simulink model "TosAeb"  (R2021a)
settings: Solver=FixedStepDiscrete  StartTime=0.0  StopTime=inf  FixedStep=0.01  RelTol=1e-3
undefined symbols (not defined in the file or in its folder): ACCModSts, AccSts1, AEB_ObjectMovingOrientation, AEB_ObjectMovingState, CN_BTCActive, CN_CurrentGearR, CN_CurrentGearR_DHT, CN_PTCActive, CN_VDCActive, CN_VSC_EspOff, CN_VSC_FCW_AEB_Error, CN_VSC_FCW_AEB_NoDispay, CN_VSC_FCW_AEB_PerfmDgdtn, CN_VSC_FCW_AEB_TempUnAvlbl, CN_VSC_VehStandstill, CNaccelAvoidMax, CNaccelAvoidMin, CNbrakePedalPress, CNcomfortStrLeftAccelMax, CNcomfortStrLeftAccelMin, CNcomfortStrRightAccelMax, CNcomfortStrRightAccelMin, CNDrvDoorOpen, CNDrvModeEco, CNDrvModeNorm, CNDrvModeOther, CNDrvModeSport, CNDrvSBRreminder, CNNull, CNobjInvalidID, CNPassDoorOpen, CNPassSBRreminder, CNPEBSAccelNegInf, CNPEBSAccelNull, CNPEBSTimeInf, CNPEBSTimeNull, CNSTOPMAX, CNSTOPNULL, CNsubjWidth, CNSwtOFF, CNSwtON, CNTTCMAX, CNTTCNULL, CNVehConfigAEBCycNone, CNVehConfigAEBNone, CNVehConfigAEBPedNone, CNVehConfigFCWNone, CNVehConfigJANone, CollisionSeverity, FALSE, FCWSnvty, JACycObjCalcInfoDefaultParams, JAPedObjCalcInfoDefaultParams, JAVehObjCalcInfoDefaultParams, latCycObjCalcInfoDefaultParams, latPedObjCalcInfoDefaultParams, longObjCalcInfoDefaultParams, ModSts, movingStatus, ObjectType ... (+1112)
libraries: simulink x3031, AEBSFunctionLib x50, Ta_Library x33, AEBSStateMachineLib x23, Mylibrary x12, simulink_extras x7, ObjSelect_lib x3, dspstat3 x2
(part 12 of 102)

== system TosAeb/FcwAebJa_Step_sys/ALG_AEBSystem/ALG_AEBOutput/AEB_EDR_JAPED_INFOMap/Subsystem ==
  JaPedTgtCalcInfo : Inport
  Action Port : ActionPort  ActionPortLabel=else
  Bus Creator : BusCreator  Inputs=25  OutDataTypeStr=Bus: AEB_EDR_LATPED_INFO_BUS
      settings: InheritFromInputs=off
  Bus Selector : BusSelector  OutputSignals=JAPedTgtObjInfo.AEBJAPedObj.FusionType,JAPedTgtObjInfo.AEBJAPedObj.ObjectID,JAPedTgtObjInfo.AEBJAPedObj.ObjectType,JAPedTgtObjInfo.AEBJAPedObj.ObjectTypeCameraConfirmed,JAPedTgtObjInfo.AEBJAPedObj.ObjectLength,JAPedTgtObjInfo.AEBJAPedObj.ObjectWidth,JAPedTgtObjInfo.AEBJAPedObj.ObjectHight,JAPedTgtObjInfo.AEBJAPedObj.ObjectYawAngle,JAPedTgtObjInfo.AEBJAPedObj.ObjectMovingState,JAPedTgtObjInfo.AEBJAPedObj.ObjectMovingOrientation,JAPedTgtObjInfo.AEBJAPedObj.dxFisrtDetection,JAPedTgtObjInfo.AEBJAPedObj.dyFisrtDetection,JAPedTgtObjInfo.AEBJAPedObj.dx,JAPedTgtObjInfo.AEBJAPedObj.dy,JAPedTgtObjInfo.AEBJAPedObj.dz,JAPedTgtObjInfo.AEBJAPedObj.vx,JAPedTgtObjInfo.AEBJAPedObj.vy,JAPedTgtObjInfo.AEBJAPedObj.ax,JAPedTgtObjInfo.AEBJAPedObj.ay,JAPedTgtObjInfo.AEBJAPedObj.dxVariance,JAPedTgtObjInfo.AEBJAPedObj.dyVariance,JAPedTgtObjInfo.AEBJAPedObj.ObjectExistProb,JAPedTgtObjInfo.AEBJAPedObj.ObjectIsObstacleProb,JAPedTgtObjInfo.AEBJAPedObj.ObjectMeasurementStatus,JAPedTgtObjInfo.AEBJAPedObj.ObjectLifeCycle
  Data Type Conversion{1..4} (4 alike) : DataTypeConversion  OutDataTypeStr=uint8
  Signal Conversion{,1..24} (25 alike) : SignalConversion  OverrideOpt=off
  AEB_EDR_JAPED_INFO : Outport  OutDataTypeStr=Bus: AEB_EDR_JAPED_INFO_BUS
  wires:
  Bus Selector/9 -> Signal Conversion8/1  "<ObjectMovingState>"
  Signal Conversion23/1 -> Bus Creator/24  "ObjectMeasurementStatus"
  Signal Conversion20/1 -> Bus Creator/11  "dxFisrtDetection"
  Bus Selector/18 -> Signal Conversion12/1  "<ax>"
  Bus Selector/23 -> Signal Conversion22/1  "<ObjectIsObstacleProb>"
  Signal Conversion13/1 -> Bus Creator/19  "ay"
  Bus Selector/25 -> Signal Conversion19/1  "<ObjectLifeCycle>"
  Bus Selector/1 -> Signal Conversion/1  "<FusionType>"
  Bus Selector/16 -> Signal Conversion15/1  "<vx>"
  Signal Conversion21/1 -> Bus Creator/22  "ObjectExistProb"
  JaPedTgtCalcInfo/1 -> Bus Selector/1
  Signal Conversion22/1 -> Bus Creator/23  "ObjectIsObstacleProb"
  Bus Selector/11 -> Signal Conversion20/1  "<dxFisrtDetection>"
  Signal Conversion10/1 -> Bus Creator/13  "dx"
  Signal Conversion4/1 -> Bus Creator/5  "ObjectLength"
  Bus Selector/7 -> Signal Conversion6/1  "<ObjectHight>"
  Bus Selector/10 -> Signal Conversion9/1  "<ObjectMovingOrientation>"
  Signal Conversion16/1 -> Bus Creator/17  "vy"
  Bus Selector/4 -> Signal Conversion3/1  "<ObjectTypeCameraConfirmed>"
  Bus Selector/5 -> Signal Conversion4/1  "<ObjectLength>"
  Bus Selector/14 -> Signal Conversion11/1  "<dy>"
  Signal Conversion1/1 -> Bus Creator/2  "ObjectID"
  Bus Selector/2 -> Signal Conversion1/1  "<ObjectID>"
  Bus Selector/19 -> Signal Conversion13/1  "<ay>"
  Signal Conversion5/1 -> Bus Creator/6  "ObjectWidth"
  Bus Selector/24 -> Signal Conversion23/1  "<ObjectMeasurementStatus>"
  Data Type Conversion1/1 -> Bus Creator/10  "ObjectMovingOrientation"
  Data Type Conversion4/1 -> Bus Creator/9  "ObjectMovingState"
  Bus Creator/1 -> AEB_EDR_JAPED_INFO/1
  Signal Conversion7/1 -> Bus Creator/8  "ObjectYawAngle"
  Bus Selector/12 -> Signal Conversion24/1  "<dyFisrtDetection>"
  Signal Conversion6/1 -> Bus Creator/7  "ObjectHight"
  Signal Conversion12/1 -> Bus Creator/18  "ax"
  Data Type Conversion2/1 -> Bus Creator/1  "FusionType"
  Bus Selector/3 -> Signal Conversion2/1  "<ObjectType>"
  Signal Conversion18/1 -> Bus Creator/21  "dyVariance"
  Signal Conversion11/1 -> Bus Creator/14  "dy"
  Signal Conversion9/1 -> Data Type Conversion1/1
  Bus Selector/8 -> Signal Conversion7/1  "<ObjectYawAngle>"
  Bus Selector/21 -> Signal Conversion18/1  "<dyVariance>"
  Data Type Conversion3/1 -> Bus Creator/3  "ObjectType"
  Signal Conversion2/1 -> Data Type Conversion3/1
  Signal Conversion15/1 -> Bus Creator/16  "vx"
  Signal Conversion3/1 -> Bus Creator/4  "ObjectTypeCameraConfirmed"
  Bus Selector/6 -> Signal Conversion5/1  "<ObjectWidth>"
  Signal Conversion24/1 -> Bus Creator/12  "dyFisrtDetection"
  Signal Conversion14/1 -> Bus Creator/15  "dz"
  Signal Conversion8/1 -> Data Type Conversion4/1
  Bus Selector/22 -> Signal Conversion21/1  "<ObjectExistProb>"
  Bus Selector/15 -> Signal Conversion14/1  "<dz>"
  Bus Selector/13 -> Signal Conversion10/1  "<dx>"
  Signal Conversion17/1 -> Bus Creator/20  "dxVariance"
  Signal Conversion19/1 -> Bus Creator/25  "ObjectLifeCycle"
  Bus Selector/17 -> Signal Conversion16/1  "<vy>"
  Signal Conversion/1 -> Data Type Conversion2/1
  Bus Selector/20 -> Signal Conversion17/1  "<dxVariance>"

== system TosAeb/FcwAebJa_Step_sys/ALG_AEBSystem/ALG_AEBOutput/AEB_EDR_JAVEH_INFOMap/If Action Subsystem ==
  Action Port : ActionPort  ActionPortLabel=if(u1)
  Bus Creator : BusCreator  Inputs=25  OutDataTypeStr=Bus: AEB_EDR_LATPED_INFO_BUS
      settings: InheritFromInputs=off
  Constant{,1..24} (25 alike) : Constant  Value=0
  Data Type Conversion{,5..24} (21 alike) : DataTypeConversion
  Data Type Conversion{1..4} (4 alike) : DataTypeConversion  OutDataTypeStr=uint8
  AEB_EDR_JAVEH_INFO : Outport  OutDataTypeStr=Bus: AEB_EDR_JAVEH_INFO_BUS
  wires:
  Constant24/1 -> Data Type Conversion24/1
  Constant18/1 -> Data Type Conversion20/1
  Constant19/1 -> Data Type Conversion21/1
  Constant21/1 -> Data Type Conversion23/1
  Constant20/1 -> Data Type Conversion22/1
  Constant16/1 -> Data Type Conversion18/1
  Constant17/1 -> Data Type Conversion19/1
  Constant23/1 -> Data Type Conversion17/1
  Constant22/1 -> Data Type Conversion16/1
  Constant8/1 -> Data Type Conversion4/1
  Constant9/1 -> Data Type Conversion1/1
  Constant11/1 -> Data Type Conversion11/1
  Constant10/1 -> Data Type Conversion10/1
  Constant14/1 -> Data Type Conversion14/1
  Constant15/1 -> Data Type Conversion15/1
  Constant13/1 -> Data Type Conversion13/1
  Constant12/1 -> Data Type Conversion12/1
  Constant4/1 -> Data Type Conversion6/1
  Constant5/1 -> Data Type Conversion7/1
  Constant7/1 -> Data Type Conversion9/1
  Constant6/1 -> Data Type Conversion8/1
  Constant2/1 -> Data Type Conversion3/1
  Constant3/1 -> Data Type Conversion5/1
  Constant1/1 -> Data Type Conversion/1
  Constant/1 -> Data Type Conversion2/1
  Bus Creator/1 -> AEB_EDR_JAVEH_INFO/1
  Data Type Conversion16/1 -> Bus Creator/17  "vy"
  Data Type Conversion9/1 -> Bus Creator/8  "ObjectYawAngle"
  Data Type Conversion22/1 -> Bus Creator/23  "ObjectIsObstacleProb"
  Data Type Conversion17/1 -> Bus Creator/18  "ax"
  Data Type Conversion11/1 -> Bus Creator/12  "dyFisrtDetection"
  Data Type Conversion19/1 -> Bus Creator/20  "dxVariance"
  Data Type Conversion15/1 -> Bus Creator/16  "vx"
  Data Type Conversion8/1 -> Bus Creator/7  "ObjectHight"
  Data Type Conversion14/1 -> Bus Creator/15  "dz"
  Data Type Conversion/1 -> Bus Creator/2  "ObjectID"
  Data Type Conversion4/1 -> Bus Creator/9  "ObjectMovingState"
  Data Type Conversion21/1 -> Bus Creator/22  "ObjectExistProb"
  Data Type Conversion3/1 -> Bus Creator/3  "ObjectType"
  Data Type Conversion1/1 -> Bus Creator/10  "ObjectMovingOrientation"
  Data Type Conversion24/1 -> Bus Creator/25  "ObjectLifeCycle"
  Data Type Conversion18/1 -> Bus Creator/19  "ay"
  Data Type Conversion6/1 -> Bus Creator/5  "ObjectLength"
  Data Type Conversion20/1 -> Bus Creator/21  "dyVariance"
  Data Type Conversion12/1 -> Bus Creator/13  "dx"
  Data Type Conversion2/1 -> Bus Creator/1  "FusionType"
  Data Type Conversion5/1 -> Bus Creator/4  "ObjectTypeCameraConfirmed"
  Data Type Conversion23/1 -> Bus Creator/24  "ObjectMeasurementStatus"
  Data Type Conversion10/1 -> Bus Creator/11  "dxFisrtDetection"
  Data Type Conversion13/1 -> Bus Creator/14  "dy"
  Data Type Conversion7/1 -> Bus Creator/6  "ObjectWidth"

== system TosAeb/FcwAebJa_Step_sys/ALG_AEBSystem/ALG_AEBOutput/AEB_EDR_JAVEH_INFOMap/Subsystem ==
  JaVehTgtCalcInfo : Inport
  Action Port : ActionPort  ActionPortLabel=else
  Bus Creator : BusCreator  Inputs=25  OutDataTypeStr=Bus: AEB_EDR_LATPED_INFO_BUS
      settings: InheritFromInputs=off
  Bus Selector : BusSelector  OutputSignals=JAVehTgtObjInfo.AEBJAVehObj.FusionType,JAVehTgtObjInfo.AEBJAVehObj.ObjectID,JAVehTgtObjInfo.AEBJAVehObj.ObjectType,JAVehTgtObjInfo.AEBJAVehObj.ObjectTypeCameraConfirmed,JAVehTgtObjInfo.AEBJAVehObj.ObjectLength,JAVehTgtObjInfo.AEBJAVehObj.ObjectWidth,JAVehTgtObjInfo.AEBJAVehObj.ObjectHight,JAVehTgtObjInfo.AEBJAVehObj.ObjectYawAngle,JAVehTgtObjInfo.AEBJAVehObj.ObjectMovingState,JAVehTgtObjInfo.AEBJAVehObj.ObjectMovingOrientation,JAVehTgtObjInfo.AEBJAVehObj.dxFisrtDetection,JAVehTgtObjInfo.AEBJAVehObj.dyFisrtDetection,JAVehTgtObjInfo.AEBJAVehObj.dx,JAVehTgtObjInfo.AEBJAVehObj.dy,JAVehTgtObjInfo.AEBJAVehObj.dz,JAVehTgtObjInfo.AEBJAVehObj.vx,JAVehTgtObjInfo.AEBJAVehObj.vy,JAVehTgtObjInfo.AEBJAVehObj.ax,JAVehTgtObjInfo.AEBJAVehObj.ay,JAVehTgtObjInfo.AEBJAVehObj.dxVariance,JAVehTgtObjInfo.AEBJAVehObj.dyVariance,JAVehTgtObjInfo.AEBJAVehObj.ObjectExistProb,JAVehTgtObjInfo.AEBJAVehObj.ObjectIsObstacleProb,JAVehTgtObjInfo.AEBJAVehObj.ObjectMeasurementStatus,JAVehTgtObjInfo.AEBJAVehObj.ObjectLifeCycle
  Data Type Conversion{1..4} (4 alike) : DataTypeConversion  OutDataTypeStr=uint8
  Signal Conversion{,1..24} (25 alike) : SignalConversion  OverrideOpt=off
  AEB_EDR_JAVEH_INFO : Outport  OutDataTypeStr=Bus: AEB_EDR_JAVEH_INFO_BUS
  wires:
  Bus Creator/1 -> AEB_EDR_JAVEH_INFO/1
  Bus Selector/18 -> Signal Conversion12/1  "<ax>"
  Bus Selector/21 -> Signal Conversion18/1  "<dyVariance>"
  Bus Selector/1 -> Signal Conversion/1  "<FusionType>"
  Signal Conversion20/1 -> Bus Creator/11  "dxFisrtDetection"
  Signal Conversion23/1 -> Bus Creator/24  "ObjectMeasurementStatus"
  Bus Selector/13 -> Signal Conversion10/1  "<dx>"
  Signal Conversion11/1 -> Bus Creator/14  "dy"
  Bus Selector/8 -> Signal Conversion7/1  "<ObjectYawAngle>"
  Bus Selector/19 -> Signal Conversion13/1  "<ay>"
  Signal Conversion3/1 -> Bus Creator/4  "ObjectTypeCameraConfirmed"
  Bus Selector/22 -> Signal Conversion21/1  "<ObjectExistProb>"
  Bus Selector/20 -> Signal Conversion17/1  "<dxVariance>"
  Signal Conversion5/1 -> Bus Creator/6  "ObjectWidth"
  Signal Conversion17/1 -> Bus Creator/20  "dxVariance"
  Signal Conversion15/1 -> Bus Creator/16  "vx"
  Signal Conversion7/1 -> Bus Creator/8  "ObjectYawAngle"
  Signal Conversion12/1 -> Bus Creator/18  "ax"
  Signal Conversion2/1 -> Data Type Conversion3/1
  Bus Selector/3 -> Signal Conversion2/1  "<ObjectType>"
  Bus Selector/14 -> Signal Conversion11/1  "<dy>"
  JaVehTgtCalcInfo/1 -> Bus Selector/1
  Bus Selector/11 -> Signal Conversion20/1  "<dxFisrtDetection>"
  Data Type Conversion2/1 -> Bus Creator/1  "FusionType"
  Bus Selector/16 -> Signal Conversion15/1  "<vx>"
  Signal Conversion10/1 -> Bus Creator/13  "dx"
  Signal Conversion18/1 -> Bus Creator/21  "dyVariance"
  Signal Conversion4/1 -> Bus Creator/5  "ObjectLength"
  Signal Conversion13/1 -> Bus Creator/19  "ay"
  Bus Selector/24 -> Signal Conversion23/1  "<ObjectMeasurementStatus>"
  Bus Selector/9 -> Signal Conversion8/1  "<ObjectMovingState>"
  Signal Conversion19/1 -> Bus Creator/25  "ObjectLifeCycle"
  Bus Selector/10 -> Signal Conversion9/1  "<ObjectMovingOrientation>"
  Data Type Conversion1/1 -> Bus Creator/10  "ObjectMovingOrientation"
  Bus Selector/2 -> Signal Conversion1/1  "<ObjectID>"
  Data Type Conversion3/1 -> Bus Creator/3  "ObjectType"
  Data Type Conversion4/1 -> Bus Creator/9  "ObjectMovingState"
  Bus Selector/12 -> Signal Conversion24/1  "<dyFisrtDetection>"
  Signal Conversion21/1 -> Bus Creator/22  "ObjectExistProb"
  Signal Conversion9/1 -> Data Type Conversion1/1
  Bus Selector/4 -> Signal Conversion3/1  "<ObjectTypeCameraConfirmed>"
  Bus Selector/5 -> Signal Conversion4/1  "<ObjectLength>"
  Signal Conversion14/1 -> Bus Creator/15  "dz"
  Signal Conversion1/1 -> Bus Creator/2  "ObjectID"
  Signal Conversion/1 -> Data Type Conversion2/1
  Signal Conversion22/1 -> Bus Creator/23  "ObjectIsObstacleProb"
  Bus Selector/23 -> Signal Conversion22/1  "<ObjectIsObstacleProb>"
  Signal Conversion8/1 -> Data Type Conversion4/1
  Bus Selector/7 -> Signal Conversion6/1  "<ObjectHight>"
  Bus Selector/15 -> Signal Conversion14/1  "<dz>"
  Bus Selector/6 -> Signal Conversion5/1  "<ObjectWidth>"
  Bus Selector/17 -> Signal Conversion16/1  "<vy>"
  Signal Conversion24/1 -> Bus Creator/12  "dyFisrtDetection"
  Signal Conversion16/1 -> Bus Creator/17  "vy"
  Signal Conversion6/1 -> Bus Creator/7  "ObjectHight"
  Bus Selector/25 -> Signal Conversion19/1  "<ObjectLifeCycle>"

== system TosAeb/FcwAebJa_Step_sys/ALG_AEBSystem/ALG_AEBOutput/AEB_EDR_LATCYC_INFOMap/If Action Subsystem ==
  Action Port : ActionPort  ActionPortLabel=if(u1)
  Bus Creator : BusCreator  Inputs=25  OutDataTypeStr=Bus: AEB_EDR_LATPED_INFO_BUS
      settings: InheritFromInputs=off
  Constant{,1..17,24..30} (25 alike) : Constant  Value=0
  Data Type Conversion{,1..3} (4 alike) : DataTypeConversion  OutDataTypeStr=uint8
  Data Type Conversion{4..24} (21 alike) : DataTypeConversion
  AEB_EDR_LATCYC_INFO : Outport  OutDataTypeStr=Bus: AEB_EDR_LATCYC_INFO_BUS
  wires:
  Constant16/1 -> Data Type Conversion16/1
  Constant17/1 -> Data Type Conversion17/1
  Constant25/1 -> Data Type Conversion19/1
  Constant24/1 -> Data Type Conversion18/1
  Constant28/1 -> Data Type Conversion22/1
  Constant29/1 -> Data Type Conversion23/1
  Constant27/1 -> Data Type Conversion21/1
  Constant26/1 -> Data Type Conversion20/1
  Constant30/1 -> Data Type Conversion24/1
  Constant8/1 -> Data Type Conversion/1
  Constant9/1 -> Data Type Conversion1/1
  Constant11/1 -> Data Type Conversion11/1
  Constant10/1 -> Data Type Conversion10/1
  Constant14/1 -> Data Type Conversion14/1
  Constant15/1 -> Data Type Conversion15/1
  Constant13/1 -> Data Type Conversion13/1
  Constant12/1 -> Data Type Conversion12/1
  Constant4/1 -> Data Type Conversion6/1
  Constant5/1 -> Data Type Conversion7/1
  Constant7/1 -> Data Type Conversion9/1
  Constant6/1 -> Data Type Conversion8/1
  Constant2/1 -> Data Type Conversion3/1
  Constant3/1 -> Data Type Conversion5/1
  Constant1/1 -> Data Type Conversion4/1
  Constant/1 -> Data Type Conversion2/1
  Data Type Conversion17/1 -> Bus Creator/18  "ax"
  Data Type Conversion23/1 -> Bus Creator/24  "ObjectMeasurementStatus"
  Data Type Conversion19/1 -> Bus Creator/20  "dxVariance"
  Data Type Conversion9/1 -> Bus Creator/8  "ObjectYawAngle"
  Data Type Conversion/1 -> Bus Creator/9  "ObjectMovingState"
  Data Type Conversion15/1 -> Bus Creator/16  "vx"
  Bus Creator/1 -> AEB_EDR_LATCYC_INFO/1
  Data Type Conversion6/1 -> Bus Creator/5  "ObjectLength"
  Data Type Conversion10/1 -> Bus Creator/11  "dxFisrtDetection"
  Data Type Conversion18/1 -> Bus Creator/19  "ay"
  Data Type Conversion12/1 -> Bus Creator/13  "dx"
  Data Type Conversion7/1 -> Bus Creator/6  "ObjectWidth"
  Data Type Conversion8/1 -> Bus Creator/7  "ObjectHight"
  Data Type Conversion11/1 -> Bus Creator/12  "dyFisrtDetection"
  Data Type Conversion16/1 -> Bus Creator/17  "vy"
  Data Type Conversion20/1 -> Bus Creator/21  "dyVariance"
  Data Type Conversion1/1 -> Bus Creator/10  "ObjectMovingOrientation"
  Data Type Conversion5/1 -> Bus Creator/4  "ObjectTypeCameraConfirmed"
  Data Type Conversion13/1 -> Bus Creator/14  "dy"
  Data Type Conversion22/1 -> Bus Creator/23  "ObjectIsObstacleProb"
  Data Type Conversion4/1 -> Bus Creator/2  "ObjectID"
  Data Type Conversion21/1 -> Bus Creator/22  "ObjectExistProb"
  Data Type Conversion24/1 -> Bus Creator/25  "ObjectLifeCycle"
  Data Type Conversion14/1 -> Bus Creator/15  "dz"
  Data Type Conversion2/1 -> Bus Creator/1  "FusionType"
  Data Type Conversion3/1 -> Bus Creator/3  "ObjectType"

== system TosAeb/FcwAebJa_Step_sys/ALG_AEBSystem/ALG_AEBOutput/AEB_EDR_LATCYC_INFOMap/Subsystem ==
  latCycTgtCalcInfo : Inport
  Action Port : ActionPort  ActionPortLabel=else
  Bus Creator : BusCreator  Inputs=25  OutDataTypeStr=Bus: AEB_EDR_LATPED_INFO_BUS
      settings: InheritFromInputs=off
  Bus Selector : BusSelector  OutputSignals=latCycObjCalcInfo.latCycObjRawInfo.FusionType,latCycObjCalcInfo.latCycObjRawInfo.ObjectID,latCycObjCalcInfo.latCycObjRawInfo.ObjectType,latCycObjCalcInfo.latCycObjRawInfo.ObjectTypeCameraConfirmed,latCycObjCalcInfo.latCycObjRawInfo.ObjectLength,latCycObjCalcInfo.latCycObjRawInfo.ObjectWidth,latCycObjCalcInfo.latCycObjRawInfo.ObjectHight,latCycObjCalcInfo.latCycObjRawInfo.ObjectYawAngle,latCycObjCalcInfo.latCycObjRawInfo.ObjectMovingState,latCycObjCalcInfo.latCycObjRawInfo.ObjectMovingOrientation,latCycObjCalcInfo.latCycObjRawInfo.dxFisrtDetection,latCycObjCalcInfo.latCycObjRawInfo.dyFisrtDetection,latCycObjCalcInfo.latCycObjRawInfo.dx,latCycObjCalcInfo.latCycObjRawInfo.dy,latCycObjCalcInfo.latCycObjRawInfo.dz,latCycObjCalcInfo.latCycObjRawInfo.vx,latCycObjCalcInfo.latCycObjRawInfo.vy,latCycObjCalcInfo.latCycObjRawInfo.ax,latCycObjCalcInfo.latCycObjRawInfo.ay,latCycObjCalcInfo.latCycObjRawInfo.dxVariance,latCycObjCalcInfo.latCycObjRawInfo.dyVariance,latCycObjCalcInfo.latCycObjRawInfo.ObjectExistProb,latCycObjCalcInfo.latCycObjRawInfo.ObjectIsObstacleProb,latCycObjCalcInfo.latCycObjRawInfo.ObjectMeasurementStatus,latCycObjCalcInfo.latCycObjRawInfo.ObjectLifeCycle
  Data Type Conversion{,1..3} (4 alike) : DataTypeConversion  OutDataTypeStr=uint8
  Signal Conversion{,1..24} (25 alike) : SignalConversion  OverrideOpt=off
  AEB_EDR_LATCYC_INFO : Outport  OutDataTypeStr=Bus: AEB_EDR_LATCYC_INFO_BUS
  wires:
  Data Type Conversion3/1 -> Bus Creator/3  "ObjectType"
  Data Type Conversion2/1 -> Bus Creator/1  "FusionType"
  Signal Conversion14/1 -> Bus Creator/15  "dz"
  Bus Selector/24 -> Signal Conversion23/1  "<ObjectMeasurementStatus>"
  Signal Conversion21/1 -> Bus Creator/22  "ObjectExistProb"
  Signal Conversion22/1 -> Bus Creator/23  "ObjectIsObstacleProb"
  Signal Conversion1/1 -> Bus Creator/2  "ObjectID"
  Signal Conversion11/1 -> Bus Creator/14  "dy"
  Bus Selector/22 -> Signal Conversion21/1  "<ObjectExistProb>"
  Signal Conversion3/1 -> Bus Creator/4  "ObjectTypeCameraConfirmed"
  Data Type Conversion1/1 -> Bus Creator/10  "ObjectMovingOrientation"
  Bus Selector/4 -> Signal Conversion3/1  "<ObjectTypeCameraConfirmed>"
  Bus Selector/25 -> Signal Conversion19/1  "<ObjectLifeCycle>"
  Bus Selector/12 -> Signal Conversion24/1  "<dyFisrtDetection>"
  Signal Conversion9/1 -> Data Type Conversion1/1
  Bus Selector/1 -> Signal Conversion/1  "<FusionType>"
  Bus Selector/19 -> Signal Conversion13/1  "<ay>"
  Signal Conversion18/1 -> Bus Creator/21  "dyVariance"
  Bus Selector/5 -> Signal Conversion4/1  "<ObjectLength>"
  Bus Selector/7 -> Signal Conversion6/1  "<ObjectHight>"
  Signal Conversion24/1 -> Bus Creator/12  "dyFisrtDetection"
  Signal Conversion6/1 -> Bus Creator/7  "ObjectHight"
  Bus Selector/2 -> Signal Conversion1/1  "<ObjectID>"
  Signal Conversion5/1 -> Bus Creator/6  "ObjectWidth"
  Signal Conversion13/1 -> Bus Creator/19  "ay"
  Signal Conversion10/1 -> Bus Creator/13  "dx"
  Signal Conversion4/1 -> Bus Creator/5  "ObjectLength"
  Signal Conversion19/1 -> Bus Creator/25  "ObjectLifeCycle"
  Bus Creator/1 -> AEB_EDR_LATCYC_INFO/1
  Bus Selector/8 -> Signal Conversion7/1  "<ObjectYawAngle>"
  Bus Selector/13 -> Signal Conversion10/1  "<dx>"
  Bus Selector/14 -> Signal Conversion11/1  "<dy>"
  Signal Conversion15/1 -> Bus Creator/16  "vx"
  Bus Selector/9 -> Signal Conversion8/1  "<ObjectMovingState>"
  Signal Conversion/1 -> Data Type Conversion2/1
  Signal Conversion8/1 -> Data Type Conversion/1
  Bus Selector/18 -> Signal Conversion12/1  "<ax>"
  Data Type Conversion/1 -> Bus Creator/9  "ObjectMovingState"
  Signal Conversion16/1 -> Bus Creator/17  "vy"
  Bus Selector/21 -> Signal Conversion18/1  "<dyVariance>"
  Signal Conversion7/1 -> Bus Creator/8  "ObjectYawAngle"
  Bus Selector/6 -> Signal Conversion5/1  "<ObjectWidth>"
  Signal Conversion17/1 -> Bus Creator/20  "dxVariance"
  Bus Selector/23 -> Signal Conversion22/1  "<ObjectIsObstacleProb>"
  Bus Selector/10 -> Signal Conversion9/1  "<ObjectMovingOrientation>"
  latCycTgtCalcInfo/1 -> Bus Selector/1
  Bus Selector/16 -> Signal Conversion15/1  "<vx>"
  Signal Conversion23/1 -> Bus Creator/24  "ObjectMeasurementStatus"
  Signal Conversion2/1 -> Data Type Conversion3/1
  Bus Selector/3 -> Signal Conversion2/1  "<ObjectType>"
  Signal Conversion20/1 -> Bus Creator/11  "dxFisrtDetection"
  Signal Conversion12/1 -> Bus Creator/18  "ax"
  Bus Selector/17 -> Signal Conversion16/1  "<vy>"
  Bus Selector/15 -> Signal Conversion14/1  "<dz>"
  Bus Selector/11 -> Signal Conversion20/1  "<dxFisrtDetection>"
  Bus Selector/20 -> Signal Conversion17/1  "<dxVariance>"

== system TosAeb/FcwAebJa_Step_sys/ALG_AEBSystem/ALG_AEBOutput/AEB_EDR_LATPED_INFOMap/If Action Subsystem ==
  Action Port : ActionPort  ActionPortLabel=if(u1)
  Bus Creator : BusCreator  Inputs=25  OutDataTypeStr=Bus: AEB_EDR_LATPED_INFO_BUS
      settings: InheritFromInputs=off
  Constant{,1..24} (25 alike) : Constant  Value=0
  Data Type Conversion{,1..3} (4 alike) : DataTypeConversion  OutDataTypeStr=uint8
  Data Type Conversion{4..24} (21 alike) : DataTypeConversion
  AEB_EDR_LATPED_INFO : Outport  OutDataTypeStr=Bus: AEB_EDR_LATPED_INFO_BUS
  wires:
  Constant23/1 -> Data Type Conversion21/1
  Constant22/1 -> Data Type Conversion24/1
  Constant24/1 -> Data Type Conversion23/1
  Constant21/1 -> Data Type Conversion18/1
  Constant20/1 -> Data Type Conversion19/1
  Constant18/1 -> Data Type Conversion20/1
  Constant19/1 -> Data Type Conversion17/1
  Constant11/1 -> Data Type Conversion10/1
  Constant10/1 -> Data Type Conversion9/1
  Constant12/1 -> Data Type Conversion12/1
  Constant13/1 -> Data Type Conversion11/1
  Constant17/1 -> Data Type Conversion14/1
  Constant16/1 -> Data Type Conversion15/1
  Constant14/1 -> Data Type Conversion16/1
  Constant15/1 -> Data Type Conversion13/1
  Constant7/1 -> Data Type Conversion22/1
  Constant6/1 -> Data Type Conversion7/1
  Constant8/1 -> Data Type Conversion/1
  Constant9/1 -> Data Type Conversion1/1
  Constant5/1 -> Data Type Conversion8/1
  Constant4/1 -> Data Type Conversion6/1
  Constant2/1 -> Data Type Conversion3/1
  Constant3/1 -> Data Type Conversion5/1
  Constant1/1 -> Data Type Conversion4/1
  Data Type Conversion23/1 -> Bus Creator/25  "ObjectLifeCycle"
  Data Type Conversion19/1 -> Bus Creator/21  "dyVariance"
  Bus Creator/1 -> AEB_EDR_LATPED_INFO/1
  Data Type Conversion6/1 -> Bus Creator/5  "ObjectLength"
  Data Type Conversion21/1 -> Bus Creator/24  "ObjectMeasurementStatus"
  Data Type Conversion17/1 -> Bus Creator/20  "dxVariance"
  Data Type Conversion13/1 -> Bus Creator/16  "vx"
  Data Type Conversion14/1 -> Bus Creator/18  "ax"
  Data Type Conversion4/1 -> Bus Creator/2  "ObjectID"
  Data Type Conversion1/1 -> Bus Creator/10  "ObjectMovingOrientation"
  Data Type Conversion/1 -> Bus Creator/9  "ObjectMovingState"
  Data Type Conversion18/1 -> Bus Creator/22  "ObjectExistProb"
  Data Type Conversion12/1 -> Bus Creator/13  "dx"
  Data Type Conversion16/1 -> Bus Creator/15  "dz"
  Data Type Conversion20/1 -> Bus Creator/19  "ay"
  Data Type Conversion7/1 -> Bus Creator/7  "ObjectHight"
  Data Type Conversion15/1 -> Bus Creator/17  "vy"
  Constant/1 -> Data Type Conversion2/1
  Data Type Conversion5/1 -> Bus Creator/4  "ObjectTypeCameraConfirmed"
  Data Type Conversion22/1 -> Bus Creator/8  "ObjectYawAngle"
  Data Type Conversion24/1 -> Bus Creator/23  "ObjectIsObstacleProb"
  Data Type Conversion8/1 -> Bus Creator/6  "ObjectWidth"
  Data Type Conversion11/1 -> Bus Creator/14  "dy"
  Data Type Conversion9/1 -> Bus Creator/11  "dxFisrtDetection"
  Data Type Conversion10/1 -> Bus Creator/12  "dyFisrtDetection"
  Data Type Conversion2/1 -> Bus Creator/1  "FusionType"
  Data Type Conversion3/1 -> Bus Creator/3  "ObjectType"

== system TosAeb/FcwAebJa_Step_sys/ALG_AEBSystem/ALG_AEBOutput/AEB_EDR_LATPED_INFOMap/Subsystem ==
  latPedTgtCalcInfo : Inport
  Action Port : ActionPort  ActionPortLabel=else
  Bus Creator : BusCreator  Inputs=25  OutDataTypeStr=Bus: AEB_EDR_LATPED_INFO_BUS
      settings: InheritFromInputs=off
  Bus Selector : BusSelector  OutputSignals=latPedObjCalcInfo.latPedObjRawInfo.FusionType,latPedObjCalcInfo.latPedObjRawInfo.ObjectID,latPedObjCalcInfo.latPedObjRawInfo.ObjectType,latPedObjCalcInfo.latPedObjRawInfo.ObjectTypeCameraConfirmed,latPedObjCalcInfo.latPedObjRawInfo.ObjectLength,latPedObjCalcInfo.latPedObjRawInfo.ObjectWidth,latPedObjCalcInfo.latPedObjRawInfo.ObjectHight,latPedObjCalcInfo.latPedObjRawInfo.ObjectYawAngle,latPedObjCalcInfo.latPedObjRawInfo.ObjectMovingState,latPedObjCalcInfo.latPedObjRawInfo.ObjectMovingOrientation,latPedObjCalcInfo.latPedObjRawInfo.dxFisrtDetection,latPedObjCalcInfo.latPedObjRawInfo.dyFisrtDetection,latPedObjCalcInfo.latPedObjRawInfo.dx,latPedObjCalcInfo.latPedObjRawInfo.dy,latPedObjCalcInfo.latPedObjRawInfo.dz,latPedObjCalcInfo.latPedObjRawInfo.vx,latPedObjCalcInfo.latPedObjRawInfo.vy,latPedObjCalcInfo.latPedObjRawInfo.ax,latPedObjCalcInfo.latPedObjRawInfo.ay,latPedObjCalcInfo.latPedObjRawInfo.dxVariance,latPedObjCalcInfo.latPedObjRawInfo.dyVariance,latPedObjCalcInfo.latPedObjRawInfo.ObjectExistProb,latPedObjCalcInfo.latPedObjRawInfo.ObjectIsObstacleProb,latPedObjCalcInfo.latPedObjRawInfo.ObjectMeasurementStatus,latPedObjCalcInfo.latPedObjRawInfo.ObjectLifeCycle
  Data Type Conversion{,1..3} (4 alike) : DataTypeConversion  OutDataTypeStr=uint8
  Signal Conversion{,1..24} (25 alike) : SignalConversion  OverrideOpt=off
  AEB_EDR_LATPED_INFO : Outport  OutDataTypeStr=Bus: AEB_EDR_LATPED_INFO_BUS
  wires:
  Bus Selector/5 -> Signal Conversion4/1  "<ObjectLength>"
  Signal Conversion24/1 -> Bus Creator/12  "dyFisrtDetection"
  Bus Selector/20 -> Signal Conversion17/1  "<dxVariance>"
  Bus Selector/18 -> Signal Conversion12/1  "<ax>"
  Bus Selector/19 -> Signal Conversion13/1  "<ay>"
  Bus Selector/10 -> Signal Conversion9/1  "<ObjectMovingOrientation>"
  Signal Conversion20/1 -> Bus Creator/11  "dxFisrtDetection"
  Data Type Conversion2/1 -> Bus Creator/1  "FusionType"
  Bus Selector/23 -> Signal Conversion22/1  "<ObjectIsObstacleProb>"
  Bus Selector/16 -> Signal Conversion15/1  "<vx>"
  Signal Conversion11/1 -> Bus Creator/14  "dy"
  Bus Selector/24 -> Signal Conversion23/1  "<ObjectMeasurementStatus>"
  Bus Selector/21 -> Signal Conversion18/1  "<dyVariance>"
  Signal Conversion22/1 -> Bus Creator/23  "ObjectIsObstacleProb"
  Signal Conversion5/1 -> Bus Creator/6  "ObjectWidth"
  Bus Selector/12 -> Signal Conversion24/1  "<dyFisrtDetection>"
  Bus Selector/11 -> Signal Conversion20/1  "<dxFisrtDetection>"
  Signal Conversion3/1 -> Bus Creator/4  "ObjectTypeCameraConfirmed"
  Bus Selector/25 -> Signal Conversion19/1  "<ObjectLifeCycle>"
  Signal Conversion9/1 -> Data Type Conversion1/1
  Signal Conversion6/1 -> Bus Creator/7  "ObjectHight"
  Bus Selector/7 -> Signal Conversion6/1  "<ObjectHight>"
  Signal Conversion10/1 -> Bus Creator/13  "dx"
  Signal Conversion13/1 -> Bus Creator/19  "ay"
  Bus Selector/3 -> Signal Conversion2/1  "<ObjectType>"
  Bus Selector/4 -> Signal Conversion3/1  "<ObjectTypeCameraConfirmed>"
  Signal Conversion14/1 -> Bus Creator/15  "dz"
  Bus Selector/8 -> Signal Conversion7/1  "<ObjectYawAngle>"
  Bus Selector/17 -> Signal Conversion16/1  "<vy>"
  Signal Conversion8/1 -> Data Type Conversion/1
  Bus Selector/2 -> Signal Conversion1/1  "<ObjectID>"
  Bus Selector/6 -> Signal Conversion5/1  "<ObjectWidth>"
  Signal Conversion21/1 -> Bus Creator/22  "ObjectExistProb"
  Data Type Conversion/1 -> Bus Creator/9  "ObjectMovingState"
  Data Type Conversion3/1 -> Bus Creator/3  "ObjectType"
  Data Type Conversion1/1 -> Bus Creator/10  "ObjectMovingOrientation"
  Signal Conversion7/1 -> Bus Creator/8  "ObjectYawAngle"
  Signal Conversion2/1 -> Data Type Conversion3/1
  Signal Conversion1/1 -> Bus Creator/2  "ObjectID"
  Bus Selector/13 -> Signal Conversion10/1  "<dx>"
  Signal Conversion15/1 -> Bus Creator/16  "vx"
  Bus Selector/1 -> Signal Conversion/1  "<FusionType>"
  Signal Conversion12/1 -> Bus Creator/18  "ax"
  Signal Conversion16/1 -> Bus Creator/17  "vy"
  Signal Conversion4/1 -> Bus Creator/5  "ObjectLength"
  Signal Conversion23/1 -> Bus Creator/24  "ObjectMeasurementStatus"
  Bus Creator/1 -> AEB_EDR_LATPED_INFO/1
  latPedTgtCalcInfo/1 -> Bus Selector/1
  Signal Conversion18/1 -> Bus Creator/21  "dyVariance"
  Signal Conversion19/1 -> Bus Creator/25  "ObjectLifeCycle"
  Bus Selector/15 -> Signal Conversion14/1  "<dz>"
  Bus Selector/9 -> Signal Conversion8/1  "<ObjectMovingState>"
  Bus Selector/14 -> Signal Conversion11/1  "<dy>"
  Signal Conversion17/1 -> Bus Creator/20  "dxVariance"
  Signal Conversion/1 -> Data Type Conversion2/1
  Bus Selector/22 -> Signal Conversion21/1  "<ObjectExistProb>"

== system TosAeb/FcwAebJa_Step_sys/ALG_AEBSystem/ALG_AEBOutput/AEB_EDR_LONOBJ_INFOMap/If Action Subsystem ==
  Action Port : ActionPort  ActionPortLabel=if(u1)
  Bus Creator : BusCreator  Inputs=24  OutDataTypeStr=Bus: AEB_EDR_LONOBJ_INFO_BUS
      settings: InheritFromInputs=off
  Constant{,1..23} (24 alike) : Constant  Value=0
  Data Type Conversion{,5..23} (20 alike) : DataTypeConversion
  Data Type Conversion{1..4} (4 alike) : DataTypeConversion  OutDataTypeStr=uint8
  AEB_EDR_LONOBJ_INFO : Outport  OutDataTypeStr=Bus: AEB_EDR_LONOBJ_INFO_BUS
  wires:
  Constant1/1 -> Data Type Conversion23/1  "ObjectID"
  Constant7/1 -> Data Type Conversion22/1  "ObjectYawAngle"
  Constant6/1 -> Data Type Conversion21/1  "ObjectHight"
  Constant5/1 -> Data Type Conversion20/1  "ObjectWidth"
  Constant4/1 -> Data Type Conversion14/1  "ObjectLength"
  Constant3/1 -> Data Type Conversion13/1  "ObjectTypeCameraConfirmed"
  Constant10/1 -> Data Type Conversion/1  "dx"
  Constant11/1 -> Data Type Conversion5/1  "dy"
  Constant12/1 -> Data Type Conversion6/1  "dz"
  Constant13/1 -> Data Type Conversion7/1  "vx"
  Constant14/1 -> Data Type Conversion8/1  "vy"
  Constant15/1 -> Data Type Conversion9/1  "ax"
  Constant16/1 -> Data Type Conversion10/1  "ay"
  Constant17/1 -> Data Type Conversion11/1  "dxVariance"
  Constant18/1 -> Data Type Conversion12/1  "dyVariance"
  Constant19/1 -> Data Type Conversion15/1  "ObjectExistProb"
  Constant20/1 -> Data Type Conversion16/1  "ObjectIsObstacleProb"
  Constant21/1 -> Data Type Conversion17/1  "ObjectMeasurementStatus"
  Constant22/1 -> Data Type Conversion18/1  "dyObject2Center"
  Constant23/1 -> Data Type Conversion19/1  "dyEgo2Center"
  Constant9/1 -> Data Type Conversion4/1  "ObjectID"
  Constant8/1 -> Data Type Conversion1/1
  Constant2/1 -> Data Type Conversion3/1
  Constant/1 -> Data Type Conversion2/1
  Data Type Conversion8/1 -> Bus Creator/15  "vy"
  Data Type Conversion5/1 -> Bus Creator/12  "dy"
  Data Type Conversion/1 -> Bus Creator/11  "dx"
  Data Type Conversion23/1 -> Bus Creator/2  "ObjectID"
  Data Type Conversion16/1 -> Bus Creator/21  "ObjectIsObstacleProb"
  Data Type Conversion6/1 -> Bus Creator/13  "dz"
  Data Type Conversion15/1 -> Bus Creator/20  "ObjectExistProb"
  Data Type Conversion12/1 -> Bus Creator/19  "dyVariance"
  Data Type Conversion7/1 -> Bus Creator/14  "vx"
  Data Type Conversion11/1 -> Bus Creator/18  "dxVariance"
  Data Type Conversion10/1 -> Bus Creator/17  "ay"
  Data Type Conversion21/1 -> Bus Creator/7  "ObjectHight"
  Data Type Conversion14/1 -> Bus Creator/5  "ObjectLength"
  Data Type Conversion1/1 -> Bus Creator/9  "ObjectMovingState"
  Data Type Conversion9/1 -> Bus Creator/16  "ax"
  Data Type Conversion4/1 -> Bus Creator/10  "ObjectMovingOrientation"
  Data Type Conversion13/1 -> Bus Creator/4  "ObjectTypeCameraConfirmed"
  Data Type Conversion22/1 -> Bus Creator/8  "ObjectYawAngle"
  Data Type Conversion20/1 -> Bus Creator/6  "ObjectWidth"
  Data Type Conversion19/1 -> Bus Creator/24  "dyEgo2Center"
  Data Type Conversion18/1 -> Bus Creator/23  "dyObject2Center"
  Data Type Conversion2/1 -> Bus Creator/1  "FusionType"
  Bus Creator/1 -> AEB_EDR_LONOBJ_INFO/1
  Data Type Conversion17/1 -> Bus Creator/22  "ObjectMeasurementStatus"
  Data Type Conversion3/1 -> Bus Creator/3  "ObjectType"

== system TosAeb/FcwAebJa_Step_sys/ALG_AEBSystem/ALG_AEBOutput/AEB_EDR_LONOBJ_INFOMap/Subsystem ==
  longObjCalcInfo : Inport
  Action Port : ActionPort  ActionPortLabel=else
  Bus Creator : BusCreator  Inputs=24  OutDataTypeStr=Bus: AEB_EDR_LONOBJ_INFO_BUS
      settings: InheritFromInputs=off
  Bus Selector : BusSelector  OutputSignals=LongObjRawInfo.FusionType,LongObjRawInfo.ObjectID,LongObjRawInfo.ObjectType,LongObjRawInfo.ObjectTypeCameraConfirmed,LongObjRawInfo.ObjectLength,LongObjRawInfo.ObjectWidth,LongObjRawInfo.ObjectHight,LongObjRawInfo.ObjectYawAngle,LongObjRawInfo.ObjectMovingState,LongObjRawInfo.ObjectMovingOrientation,LongObjRawInfo.dx,LongObjRawInfo.dy,LongObjRawInfo.dz,LongObjRawInfo.vx,LongObjRawInfo.vy,LongObjRawInfo.ax,LongObjRawInfo.ay,LongObjRawInfo.dxVariance,LongObjRawInfo.dyVariance,LongObjRawInfo.ObjectExistProb,LongObjRawInfo.ObjectIsObstacleProb,LongObjRawInfo.ObjectMeasurementStatus,LongObjRawInfo.dyObject2Center,LongObjRawInfo.dyEgo2Center
  Data Type Conversion{1..4} (4 alike) : DataTypeConversion  OutDataTypeStr=uint8
  Signal Conversion{,1..23} (24 alike) : SignalConversion  OverrideOpt=off
  AEB_EDR_LONOBJ_INFO : Outport  OutDataTypeStr=Bus: AEB_EDR_LONOBJ_INFO_BUS
  wires:
  Bus Selector/9 -> Signal Conversion8/1  "<ObjectMovingState>"
  Bus Selector/11 -> Signal Conversion10/1  "<dx>"
  Bus Selector/3 -> Signal Conversion2/1  "<ObjectType>"
  Data Type Conversion2/1 -> Bus Creator/1  "FusionType"
  Signal Conversion2/1 -> Data Type Conversion3/1
  Signal Conversion19/1 -> Bus Creator/24  "dyEgo2Center"
  Bus Creator/1 -> AEB_EDR_LONOBJ_INFO/1
  Signal Conversion7/1 -> Bus Creator/8  "ObjectYawAngle"
  Bus Selector/21 -> Signal Conversion22/1  "<ObjectIsObstacleProb>"
  Signal Conversion12/1 -> Bus Creator/16  "ax"
  Data Type Conversion1/1 -> Bus Creator/9  "ObjectMovingState"
  Signal Conversion6/1 -> Bus Creator/7  "ObjectHight"
  Bus Selector/24 -> Signal Conversion19/1  "<dyEgo2Center>"
  Data Type Conversion4/1 -> Bus Creator/10  "ObjectMovingOrientation"
  Bus Selector/23 -> Signal Conversion23/1  "<dyObject2Center>"
  Signal Conversion21/1 -> Bus Creator/20  "ObjectExistProb"
  Signal Conversion8/1 -> Data Type Conversion1/1
  Bus Selector/13 -> Signal Conversion14/1  "<dz>"
  Bus Selector/14 -> Signal Conversion15/1  "<vx>"
  Signal Conversion17/1 -> Bus Creator/18  "dxVariance"
  longObjCalcInfo/1 -> Bus Selector/1
  Data Type Conversion3/1 -> Bus Creator/3  "ObjectType"
  Signal Conversion1/1 -> Bus Creator/2  "ObjectID"
  Signal Conversion22/1 -> Bus Creator/21  "ObjectIsObstacleProb"
  Bus Selector/8 -> Signal Conversion7/1  "<ObjectYawAngle>"
  Bus Selector/17 -> Signal Conversion13/1  "<ay>"
  Bus Selector/12 -> Signal Conversion11/1  "<dy>"
  Bus Selector/20 -> Signal Conversion21/1  "<ObjectExistProb>"
  Signal Conversion4/1 -> Bus Creator/5  "ObjectLength"
  Bus Selector/1 -> Signal Conversion/1  "<FusionType>"
  Signal Conversion14/1 -> Bus Creator/13  "dz"
  Bus Selector/22 -> Signal Conversion20/1  "<ObjectMeasurementStatus>"
  Signal Conversion3/1 -> Bus Creator/4  "ObjectTypeCameraConfirmed"
  Signal Conversion11/1 -> Bus Creator/12  "dy"
  Signal Conversion13/1 -> Bus Creator/17  "ay"
  Signal Conversion18/1 -> Bus Creator/19  "dyVariance"
  Bus Selector/18 -> Signal Conversion17/1  "<dxVariance>"
  Signal Conversion10/1 -> Bus Creator/11  "dx"
  Bus Selector/6 -> Signal Conversion5/1  "<ObjectWidth>"
  Bus Selector/5 -> Signal Conversion4/1  "<ObjectLength>"
  Signal Conversion5/1 -> Bus Creator/6  "ObjectWidth"
  Signal Conversion9/1 -> Data Type Conversion4/1
  Signal Conversion16/1 -> Bus Creator/15  "vy"
  Signal Conversion23/1 -> Bus Creator/23  "dyObject2Center"
  Bus Selector/16 -> Signal Conversion12/1  "<ax>"
  Bus Selector/4 -> Signal Conversion3/1  "<ObjectTypeCameraConfirmed>"
  Bus Selector/19 -> Signal Conversion18/1  "<dyVariance>"
  Bus Selector/7 -> Signal Conversion6/1  "<ObjectHight>"
  Signal Conversion20/1 -> Bus Creator/22  "ObjectMeasurementStatus"
  Signal Conversion15/1 -> Bus Creator/14  "vx"
  Bus Selector/10 -> Signal Conversion9/1  "<ObjectMovingOrientation>"
  Bus Selector/15 -> Signal Conversion16/1  "<vy>"
  Signal Conversion/1 -> Data Type Conversion2/1
  Bus Selector/2 -> Signal Conversion1/1  "<ObjectID>"

== system TosAeb/AEB_Objsel/TA_InfoRebuild/TAObjInfoCalc/For Each Subsystem/Info_rebuild/Basic_Info ==
  Obj_Infomation : Inport
  Abs : Abs
  Atan : Trigonometry  Operator=atan
  Bus Selector : BusSelector  OutputSignals=VelX_s,VelY_s,AccX_s,AccY_s,PosX_s,PosY_s
  Compare To Constant : simulink/Logic and Bit Operations/Compare To Constant  relop=<=  const=0.001  OutDataTypeStr=boolean
  Constant{,1..3,5} (5 alike) : Constant  Value=0  OutDataTypeStr=single
  Constant4 : Constant  Value=0.001  OutDataTypeStr=single
  Divide : Product  Inputs=*/
  Switch : Switch  Criteria=u2 > Threshold
  vxRel : Outport
  vyRel : Outport  Port=2
  vxRelStd : Outport  Port=3
  vyRelStd : Outport  Port=4
  axRel : Outport  Port=5
  ayRel : Outport  Port=6
  axRelStd : Outport  Port=7
  ayRelStd : Outport  Port=8
  azimuthAngle : Outport  Port=9
  azimuthAngleStd : Outport  Port=10
  wires:
  Obj_Infomation/1 -> Bus Selector/1
  Bus Selector/1 -> [VelX]  "<VelX_s>"
  Bus Selector/2 -> [VelY]  "<VelY_s>"
  Bus Selector/3 -> [AccX]  "<AccX_s>"
  Bus Selector/4 -> [AccY]  "<AccY_s>"
  Bus Selector/5 -> [PosX]  "<PosX_s>"
  Bus Selector/6 -> [PosY]  "<PosY_s>"
  [VelX] -> vxRel/1
  [VelY] -> vyRel/1
  Constant/1 -> vxRelStd/1
  Constant1/1 -> vyRelStd/1
  [AccX] -> axRel/1
  [AccY] -> ayRel/1
  Constant2/1 -> axRelStd/1
  Constant3/1 -> ayRelStd/1
  [PosX] -> Switch/3, Abs/1
  Compare To Constant/1 -> Switch/2
  Constant4/1 -> Switch/1
  Abs/1 -> Compare To Constant/1
  [PosY] -> Divide/1
  Switch/1 -> Divide/2
  Divide/1 -> Atan/1
  Atan/1 -> azimuthAngle/1
  Constant5/1 -> azimuthAngleStd/1

== system TosAeb/AEB_Objsel/TA_InfoRebuild/TAObjInfoCalc/For Each Subsystem/Info_rebuild/Road_relative ==
  Obj_Infomation : Inport
  Road_Info : Inport  Port=2
  LineInfo_Process : Subsystem
  RebuildInfo_Process : Subsystem
  objLatDist2LaneCenter : Outport
  objLatDist2EgoCenter : Outport  Port=2
  objPosition : Outport  Port=3
  wires:
  Road_Info/1 -> LineInfo_Process/1(Road_Info)
  Obj_Infomation/1 -> RebuildInfo_Process/1(Obj_Infomation)
  LineInfo_Process/1(LeftLine_Info) -> RebuildInfo_Process/2(LeftLine_Info)
  LineInfo_Process/2(RightLine_Info) -> RebuildInfo_Process/3(RightLine_Info)
  RebuildInfo_Process/2(objLatDist2EgoCenter) -> objLatDist2EgoCenter/1
  RebuildInfo_Process/1(objLatDist2LaneCenter) -> objLatDist2LaneCenter/1
  RebuildInfo_Process/3(objPosition) -> objPosition/1

== system TosAeb/AEB_Objsel/TA_Processor/AEB_ObjSelect/TargetInfoCheck/Data_precess/ObjInfo_Assignment ==
  Overlap_Flg : Inport
  Boudingbox_Calculate : Inport  Port=2
  Obj_Coef : Inport  Port=3
  TTI : Inport  Port=4
  LatObj_Valid : Inport  Port=5
  Lat_ObjIntersect : Inport  Port=6
  Lat_TTR : Inport  Port=7
  JA_TTR : Inport  Port=8
  Signal Conversion{,1..7} (8 alike) : SignalConversion  OverrideOpt=off
  Boudingbox_CalculateAssign : Outport
  Obj_CoefAssign : Outport  Port=2
  TTIAssign : Outport  Port=3
  LatObj_ValidAssign : Outport  Port=4
  Lat_ObjIntersectAssign : Outport  Port=5
  Lat_TTRAssign : Outport  Port=6
  Overlap_FlgAssign : Outport  Port=7
  JA_TTRAssign : Outport  Port=8
  wires:
  Signal Conversion6/1 -> Lat_TTRAssign/1
  Signal Conversion/1 -> Overlap_FlgAssign/1
  Signal Conversion4/1 -> LatObj_ValidAssign/1
  Signal Conversion1/1 -> Boudingbox_CalculateAssign/1
  Signal Conversion5/1 -> Lat_ObjIntersectAssign/1
  Signal Conversion2/1 -> Obj_CoefAssign/1
  Signal Conversion3/1 -> TTIAssign/1
  Overlap_Flg/1 -> Signal Conversion/1
  Boudingbox_Calculate/1 -> Signal Conversion1/1
  Obj_Coef/1 -> Signal Conversion2/1
  TTI/1 -> Signal Conversion3/1
  LatObj_Valid/1 -> Signal Conversion4/1
  Lat_ObjIntersect/1 -> Signal Conversion5/1
  Lat_TTR/1 -> Signal Conversion6/1
  JA_TTR/1 -> Signal Conversion7/1
  Signal Conversion7/1 -> JA_TTRAssign/1

== system TosAeb/AEB_Objsel/TA_Processor/AEB_ObjSelect/TargetInfoCheck/Data_precess/ObjInfo_Select ==
  Index : Inport
  TA_ObjID : Inport  Port=2
  TA_ObjType : Inport  Port=3
  TA_ObjState : Inport  Port=4
  TA_ObjOrientation : Inport  Port=5
  TA_ObjfusType : Inport  Port=6
  TA_ObjLaneAssign : Inport  Port=7
  TA_ObjDx : Inport  Port=8
  TA_ObjDy : Inport  Port=9
  TA_ObjDxStd : Inport  Port=10
  TA_ObjDyStd : Inport  Port=11
  TA_ObjVx : Inport  Port=12
  TA_ObjVy : Inport  Port=13
  TA_ObjVxStd : Inport  Port=14
  TA_ObjVyStd : Inport  Port=15
  TA_ObjVxAbs : Inport  Port=16
  TA_ObjVyAbs : Inport  Port=17
  TA_ObjLength : Inport  Port=18
  TA_ObjWidth : Inport  Port=19
  TA_ObjHight : Inport  Port=20
  TA_ObjAx : Inport  Port=21
  TA_ObjAy : Inport  Port=22
  TA_ObjAxAbs : Inport  Port=23
  TA_ObjAyAbs : Inport  Port=24
  TA_ObjConfidence : Inport  Port=25
  TA_ObjCenterAngle : Inport  Port=26
  TA_ObjHeadAngle : Inport  Port=27
  TA_ObjHeadAngleStd : Inport  Port=28
  Bus Creator : BusCreator  Inputs=27
  Index Vector{,1..25} (26 alike) : MultiPortSwitch  DataPortOrder=Zero-based contiguous  Inputs=1  zeroidx=on
  Index Vector26 : MultiPortSwitch  DataPortOrder=Zero-based contiguous  Inputs=1  zeroidx=on  OutDataTypeStr=single
  TA_ObsInfo : Outport
  wires:
  TA_ObjVxStd/1 -> Index Vector12/2
  TA_ObjDy/1 -> Index Vector7/2
  Index Vector4/1 -> Bus Creator/5  "TA_ObsfusType"
  Index Vector24/1 -> Bus Creator/19  "TA_ObsHight"
  Index Vector22/1 -> Bus Creator/17  "TA_ObsLength"
  Index Vector11/1 -> Bus Creator/12  "TA_ObsVy"
  Index Vector25/1 -> Bus Creator/20  "TA_ObsAx"
  Index Vector14/1 -> Bus Creator/15  "TA_ObsVxAbs"
  TA_ObjAx/1 -> Index Vector25/2
  TA_ObjID/1 -> Index Vector/2
  Index Vector15/1 -> Bus Creator/16  "TA_ObsVyAbs"
  TA_ObjType/1 -> Index Vector1/2
  Index Vector5/1 -> Bus Creator/6  "TA_ObsLaneAssign"
  TA_ObjAxAbs/1 -> Index Vector16/2
  Index Vector7/1 -> Bus Creator/8  "TA_ObsDy"
  TA_ObjVyStd/1 -> Index Vector13/2
  TA_ObjVyAbs/1 -> Index Vector15/2
  TA_ObjVxAbs/1 -> Index Vector14/2
  TA_ObjDx/1 -> Index Vector6/2
  TA_ObjAyAbs/1 -> Index Vector17/2
  Index Vector3/1 -> Bus Creator/4  "TA_ObsOrientation"
  Index Vector23/1 -> Bus Creator/18  "TA_ObsWidth"
  TA_ObjVy/1 -> Index Vector11/2
  Index Vector1/1 -> Bus Creator/2  "TA_ObsType"
  Index Vector26/1 -> Bus Creator/21  "TA_ObsAy"
  TA_ObjAy/1 -> Index Vector26/2
  TA_ObjConfidence/1 -> Index Vector18/2
  Index Vector10/1 -> Bus Creator/11  "TA_ObsVx"
  Index Vector2/1 -> Bus Creator/3  "TA_ObsState"
  TA_ObjfusType/1 -> Index Vector4/2
  TA_ObjDxStd/1 -> Index Vector8/2
  TA_ObjHeadAngleStd/1 -> Index Vector21/2
  Index Vector12/1 -> Bus Creator/13  "TA_ObsVxStd"
  Index Vector13/1 -> Bus Creator/14  "TA_ObsVyStd"
  Index Vector19/1 -> Bus Creator/25  "TA_ObsCenterAngle"
  TA_ObjCenterAngle/1 -> Index Vector19/2
  TA_ObjState/1 -> Index Vector2/2
  TA_ObjLength/1 -> Index Vector22/2
  TA_ObjVx/1 -> Index Vector10/2
  Index Vector20/1 -> Bus Creator/26  "TA_ObsHeadAngle"
  TA_ObjOrientation/1 -> Index Vector3/2
  Index Vector9/1 -> Bus Creator/10  "TA_ObsDyStd"
  TA_ObjLaneAssign/1 -> Index Vector5/2
  Index Vector21/1 -> Bus Creator/27  "TA_ObsHeadAngleStd"
  Index Vector18/1 -> Bus Creator/24  "TA_ObsConfidence"
  TA_ObjWidth/1 -> Index Vector23/2
  Index Vector6/1 -> Bus Creator/7  "TA_ObsDx"
  TA_ObjHight/1 -> Index Vector24/2
  Index Vector8/1 -> Bus Creator/9  "TA_ObsDxStd"
  TA_ObjDyStd/1 -> Index Vector9/2
  TA_ObjHeadAngle/1 -> Index Vector20/2
  Bus Creator/1 -> TA_ObsInfo/1
  Index/1 -> Index Vector/1, Index Vector1/1, Index Vector2/1, Index Vector3/1, Index Vector4/1, Index Vector5/1, Index Vector6/1, Index Vector7/1, Index Vector8/1, Index Vector9/1, Index Vector10/1, Index Vector11/1, Index Vector12/1, Index Vector13/1, Index Vector14/1, Index Vector15/1, Index Vector22/1, Index Vector23/1, Index Vector24/1, Index Vector25/1, Index Vector26/1, Index Vector16/1, Index Vector17/1, Index Vector18/1, Index Vector19/1, Index Vector20/1, Index Vector21/1
  Index Vector17/1 -> Bus Creator/23  "TA_ObsAyAbs"
  Index Vector16/1 -> Bus Creator/22  "TA_ObsAxAbs"
  Index Vector/1 -> Bus Creator/1  "TA_ObsID"

== system TosAeb/AEB_Objsel/TA_Processor/AEB_ObjSelect/TargetInfoCheck/Data_precess/TA_EffectCalculate ==
  TA_ObsInfo : Inport
  VehSelfData : Inport  Port=2
  PathEstimn : Inport  Port=3
  Obj_FilterFlgCalculate : Subsystem
  ValideateObj : Subsystem
  Overlap_Flg : Outport
  Boudingbox_Calculate : Outport  Port=2
  Obj_Coef : Outport  Port=3
  TTI : Outport  Port=4
  LatObj_Valid : Outport  Port=5
  Lat_ObjIntersect : Outport  Port=6
  Lat_TTR : Outport  Port=7
  JA_TTR : Outport  Port=8
  Utility : Outport  Port=9
  Meas_WeightCoef_1 : Outport  Port=10
  Meas_WeightCoef_2 : Outport  Port=11
  wires:
  TA_ObsInfo/1 -> ValideateObj/1(TA_ObsInfo), [TA_ObsInfo]
  VehSelfData/1 -> ValideateObj/2(VehSelfData), [VehSelfData]
  PathEstimn/1 -> ValideateObj/3(PathEstimn), [PathEstimn]
  ValideateObj/3(Overlap_Prediction) -> Obj_FilterFlgCalculate/3(Overlap_Prediction)
  ValideateObj/2(Overlap_Current) -> Obj_FilterFlgCalculate/2(Overlap_Current)
  ValideateObj/1(Utility) -> Utility/1, Obj_FilterFlgCalculate/1(Utility)
  [PathEstimn] -> Obj_FilterFlgCalculate/4(PahEstimn)
  [TA_ObsInfo] -> Obj_FilterFlgCalculate/5(TA_ObjInfo)
  [VehSelfData] -> Obj_FilterFlgCalculate/6(VehSelfData)
  Obj_FilterFlgCalculate/2(Boudingbox_Calculate) -> Boudingbox_Calculate/1
  Obj_FilterFlgCalculate/1(Overlap_Flg) -> Overlap_Flg/1
  Obj_FilterFlgCalculate/3(Obj_Coef) -> Obj_Coef/1
  Obj_FilterFlgCalculate/4(TTI) -> TTI/1
  Obj_FilterFlgCalculate/5(LatObj_Valid) -> LatObj_Valid/1
  Obj_FilterFlgCalculate/6(Lat_ObjIntersect) -> Lat_ObjIntersect/1
  Obj_FilterFlgCalculate/7(Lat_TTR) -> Lat_TTR/1
  Obj_FilterFlgCalculate/8(JA_TTR) -> JA_TTR/1
  Obj_FilterFlgCalculate/9(Meas_WeightCoef_1) -> Meas_WeightCoef_1/1
  Obj_FilterFlgCalculate/10(Meas_WeightCoef_2) -> Meas_WeightCoef_2/1

== system TosAeb/AEB_Objsel/TA_Processor/AEB_ObjSelect/TargetSelect/Lat_ObjSelect/AEB_LatObj ==
  Lat_ObjNum : Inport
  LatObj_Valid : Inport  Port=2
  TA_ObjInfo : Inport  Port=3
  Lat_ObjIntersect : Inport  Port=4
  Lat_TTR : Inport  Port=5
  AEB_LatTAelse : Subsystem  TreatAsAtomicUnit=on
  AEB_LatTAif : Subsystem  TreatAsAtomicUnit=on
  If : If
  Merge : Merge
  Merge1 : Merge
  ObjNumValid : Subsystem
  Lat_ObjSortNum : Outport
  Lat_ObjID : Outport  Port=2
  wires:
  Lat_ObjNum/1 -> ObjNumValid/1(Lat_ObjNum)
  ObjNumValid/1(Obj_NumBelow) -> If/1
  If/1 -> AEB_LatTAif/Action
  TA_ObjInfo/1 -> AEB_LatTAif/2(TA_ObjInfo), AEB_LatTAelse/2(TA_ObjInfo)
  If/2 -> AEB_LatTAelse/Action
  LatObj_Valid/1 -> AEB_LatTAelse/1(LatObj_Valid), AEB_LatTAif/1(LatObj_Valid)
  Lat_ObjIntersect/1 -> AEB_LatTAelse/3(Lat_ObjIntersect)
  Lat_TTR/1 -> AEB_LatTAelse/4(Lat_TTR)
  AEB_LatTAif/1(num) -> Merge/1
  AEB_LatTAelse/1(num) -> Merge/2
  AEB_LatTAif/2(ID) -> Merge1/1
  AEB_LatTAelse/2(ID) -> Merge1/2
  Merge1/1 -> Lat_ObjID/1
  Merge/1 -> Lat_ObjSortNum/1
